simulink model "ospfb_2x_64kc_8i_16o"  (R2021a)
settings: Solver=VariableStepAuto  StartTime=0.0  StopTime=2304  RelTol=1e-3
undefined symbols (not defined in the file or in its folder): pfb_coeff_gen_calc
libraries: xbsIndex_r4 x37193, casper_library_misc x226, casper_library_delays x184
(part 187 of 313)

== system ospfb_2x_64kc_8i_16o/fft2/fft_direct/butterfly3_0/twiddle/coeff_gen/cosin ==
  theta : Inport
  misci : Inport  Port=2
  Constant4 : xbsIndex_r4/Constant  const=0  gui_display_data_type=Fixed-point  arith_type=Unsigned  n_bits=1  bin_pt=0  preci_type=Single  exp_width=8  frac_width=24  explicit_period=on  period=1  equ=P=C  opselect=C  inp2=PCIN>>17  opr=+  inp1=P  carry=CIN  dbl_ovrd=off  block_type=constant
  Constant5 : xbsIndex_r4/Constant  const=0  gui_display_data_type=Fixed-point  arith_type=Unsigned  n_bits=1  bin_pt=0  preci_type=Single  exp_width=8  frac_width=24  explicit_period=on  period=1  equ=P=C  opselect=C  inp2=PCIN>>17  opr=+  inp1=P  carry=CIN  dbl_ovrd=off  block_type=constant
  Delay : xbsIndex_r4/Delay  rst=off  infoeditControl=Selection of Reset will increase slice count due to use of real FFs and instead of SRLs  en=off  latency=bram_latency  reg_retiming=on  dbl_ovrd=off  block_type=delay
  Delay10 : xbsIndex_r4/Delay  rst=off  infoeditControl=Selection of Reset will increase slice count due to use of real FFs and instead of SRLs  en=off  latency=bram_latency  reg_retiming=on  dbl_ovrd=off  block_type=delay
  Delay8 : xbsIndex_r4/Delay  rst=off  infoeditControl=Selection of Reset will increase slice count due to use of real FFs and instead of SRLs  en=off  latency=bram_latency  reg_retiming=on  dbl_ovrd=off  block_type=delay
  ROM : xbsIndex_r4/ROM  depth=2^(12)  initVector=[34359476224 34359738355 34359738343 34359738330 34359738318 34359738305 34359738293 34359738280 34359738267 34359738255 34359738242 34359738230 34359738217 34359738205 34359738192 34359738180 34359738167 34359738154 34359738142 34359738129 34359738117 34359738104 34359738092 34359738079 34359738066 34359738054 34359738041 34359738029 34359738016 34359738004 34359737991 34359737978 34359737966 34359737953 34359737941 34359737928 34359737916 34359737903 34359737890 34359737878 34359737865 34359737853 34359737840 34359737828 34359737815 34359737803 34359737790 34359737777 34359737765 34359737752 34359475596 34359475583 34359475571 34359475558 34359475545 34359475533 34359475520 34359475508 34359475495 34359475483 34359475470 34359475457 34359475445 34359475432 34359475420 34359213263 34359213251 34359213238 34359213225 34359213213 34359213200 34359213188 34359213175 34359213163 34359213150 34359213138 34359213125 34358950968 34358950956 34358950943 34358950931 34358950918 34358950906 34358950893 34358950880 34358950868 34358950855 34358688699 34358688686 34358688674 34358688661 34358688648 34358688636 34358688623 34358688611 34358688598 34358426442 34358426429 34358426417 34358426404 34358426391 34358426379 34358426366 34358426354 34358164197 34358164185 34358164172 34358164159 34358164147 34358164134 34358164122 34358164109 34357901953 34357901940 34357901927 34357901915 34357901902 34357901890 34357901877 34357639721 34357639708 34357639696 34357639683 34357639670 34357639658 34357639645 34357377489 34357377476 34357377464 34357377451 34357377438 34357377426 34357377413 34357115257 34357115244 34357115232 34357115219 34357115206 34357115194 34356853037 34356853025 34356853012 34356853000 34356852987 34356852975 34356590818 34356590805 34356590793 34356590780 34356590768 34356328611 34356328599 34356328586 34356328573 34356328561 34356328548 34356066392 34356066379 34356066367 34356066354 34356066341 34355804185 34355804172 34355804160 34355804147 34355804135 34355541978 34355541966 34355541953 34355541940 34355541928 34355279771 34355279759 34355279746 34355279734 34355279721 34355017564 34355017552 34355017539 34355017527 34354755370 34354755358 34354755345 34354755332 34354755320 34354493163 34354493151 34354493138 34354493126 34354230969 34354230957 34354230944 34354230931 34354230919 34353968762 34353968750 34353968737 34353968725 34353706568 34353706555 34353706543 34353706530 34353444374 34353444361 34353444349 34353444336 34353182179 34353182167 34353182154 34353182142 34352919985 34352919973 34352919960 34352919948 34352657791 34352657778 34352657766 34352657753 34352395597 34352395584 34352395572 34352395559 34352133402 34352133390 34352133377 34352133365 34351871208 34351871196 34351871183 34351609027 34351609014 34351609001 34351608989 34351346832 34351346820 34351346807 34351084651 34351084638 34351084625 34351084613 34350822456 34350822444 34350822431 34350560275 34350560262 34350560250 34350560237 34350298080 34350298068 34350298055 34350035899 34350035886 34350035874 34349773717 34349773704 34349773692 34349773679 34349511523 34349511510 34349511498 34349249341 34349249329 34349249316 34348987159 34348987147 34348987134 34348724978 34348724965 34348724953 34348462796 34348462783 34348462771 34348200614 34348200602 34348200589 34347938433 34347938420 34347938408 34347676251 34347676238 34347676226 34347414069 34347414057 34347414044 34347151888 34347151875 34347151862 34346889706 34346889693 34346889681 34346627524 34346627512 34346627499 34346365343 34346365330 34346365317 34346103161 34346103148 34346103136 34345840979 34345840967 34345578810 34345578797 34345578785 34345316628 34345316616 34345316603 34345054447 34345054434 34344792278 34344792265 34344792252 34344530096 34344530083 34344530071 34344267914 34344267902 34344005745 34344005733 34344005720 34343743563 34343743551 34343743538 34343481382 34343481369 34343219213 34343219200 34343219187 34342957031 34342957018 34342694862 34342694849 34342694837 ...(+45156 chars)]  distributed_mem=Block RAM  rst=off  init_reg=0  en=off  latency=bram_latency  gui_display_data_type=Fixed-point  arith_type=Unsigned  n_bits=n_bits*2  bin_pt=0  preci_type=Single  exp_width=8  frac_width=24  optimize=Speed  use_rpm=on  dbl_ovrd=off  simulation_type=fast_sim  block_type=sprom
  Terminator : Terminator
  Terminator1 : Terminator
  Terminator4 : Terminator
  add_convert0 : Subsystem
  add_convert1 : Subsystem
  assert : xbsIndex_r4/Assert  assert_type=on  type_source=Explicitly  gui_display_data_type=Fixed-point  arith_type=Unsigned  n_bits=12  bin_pt=0  preci_type=Single  exp_width=8  frac_width=24  assert_rate=off  rate_source=Explicitly  period=1  output_port=on  block_type=assert
  c_to_ri : casper_library_misc/c_to_ri  n_bits=n_bits  bin_pt=bin_pt
      settings: SourceProductName=CASPER DSP Blockset
  invert0 : Subsystem  mask{n_bits=n_bits, bin_pt=bin_pt, neg_latency=neg_latency, mux_latency=mux_latency}
      mask prompts: n_bits "bit width"; bin_pt "binary point"; neg_latency "negate latency"; mux_latency "mux latency"
  invert1 : Subsystem  mask{n_bits=n_bits, bin_pt=bin_pt, neg_latency=neg_latency, mux_latency=mux_latency}
      mask prompts: n_bits "bit width"; bin_pt "binary point"; neg_latency "negate latency"; mux_latency "mux latency"
  cos : Outport
  -sin : Outport  Port=2
  misco : Outport  Port=3
  wires:
  theta/1 -> assert/1
  assert/1 -> add_convert0/1(theta), add_convert1/1(theta)
  Constant4/1 -> add_convert0/2(misci)
  add_convert0/3(misco) -> Terminator/1
  misci/1 -> add_convert1/2(misci)
  add_convert1/3(misco) -> Delay/1
  add_convert0/2(add) -> ROM/1
  add_convert1/2(add) -> Terminator4/1
  ROM/1 -> c_to_ri/1
  add_convert1/1(negate) -> Delay8/1
  add_convert0/1(negate) -> Delay10/1
  Delay10/1 -> invert0/1(negate)
  Constant5/1 -> invert0/3(misci)
  c_to_ri/1 -> invert0/2(in)
  Delay8/1 -> invert1/1(negate)
  c_to_ri/2 -> invert1/2(in)
  invert0/2(misco) -> Terminator1/1
  Delay/1 -> invert1/3(misci)
  invert0/1(out) -> cos/1
  invert1/1(out) -> -sin/1
  invert1/2(misco) -> misco/1
